simulink model "MemMgrForClima"  (R2022b)
settings: Solver=FixedStepDiscrete  StartTime=0.0  StopTime=10.0  FixedStep=0.1  RelTol=1e-3
model workspace (MAT data in the model file):
  MemMgrForClimaNvArray : Simulink.Signal (value not decoded)
  MemMgrForClimaNvInit : Simulink.Parameter (value not decoded)
symbols that are objects in the model workspace (value not decoded): MemMgrForClimaNvInit
(part 2 of 2)

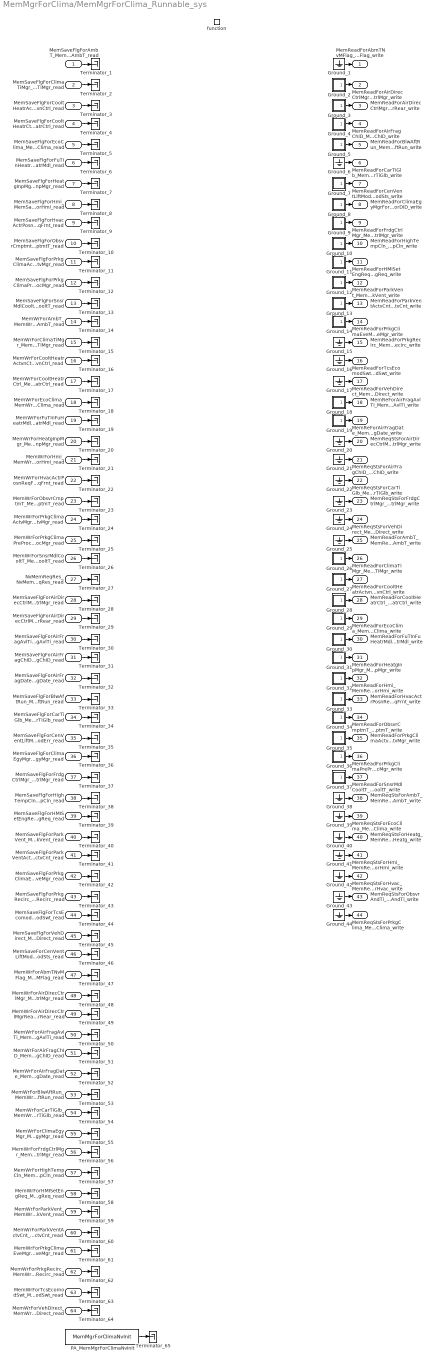
== system MemMgrForClima/MemMgrForClima_Runnable_sys ==
  MemSaveFlgForAmbT_MemSaveFlgForAmbT_read : Inport
  MemSaveFlgForClimaTiMgr_MemSaveFlgForClimaTiMgr_read : Inport  Port=2
  MemSaveFlgForCooltHeatrActvnCtrl_MemSaveFlgForCooltHeatrActvnCtrl_read : Inport  Port=3
  MemSaveFlgForCooltHeatrCtrl_MemSaveFlgForCooltHeatrCtrl_read : Inport  Port=4
  MemSaveFlgForEcoClima_MemSaveFlgForEcoClima_read : Inport  Port=5
  MemSaveFlgForFuTInHeatrMdl_MemSaveFlgForFuTInHeatrMdl_read : Inport  Port=6
  MemSaveFlgForHeatgInpMgr_MemSaveFlgForHeatgInpMgr_read : Inport  Port=7
  MemSaveFlgForHmi_MemSaveFlgForHmi_read : Inport  Port=8
  MemSaveFlgForHvacActrPosnReqFrnt_MemSaveFlgForHvacActrPosnReqFrnt_read : Inport  Port=9
  MemSaveFlgForObsvrCmptmtT_MemSaveFlgForObsvrCmptmtT_read : Inport  Port=10
  MemSaveFlgForPrkgClimaActvMgr_MemSaveFlgForPrkgClimaActvMgr_read : Inport  Port=11
  MemSaveFlgForPrkgClimaPreProcMgr_MemSaveFlgForPrkgClimaPreProcMgr_read : Inport  Port=12
  MemSaveFlgForSnsrMdlCooltT_MemSaveFlgForSnsrMdlCooltT_read : Inport  Port=13
  MemWrForAmbT_MemWrForAmbT_read : Inport  Port=14
  MemWrForClimaTiMgr_MemWrForClimaTiMgr_read : Inport  Port=15
  MemWrForCooltHeatrActvnCtrl_MemWrForCooltHeatrActvnCtrl_read : Inport  Port=16
  MemWrForCooltHeatrCtrl_MemWrForCooltHeatrCtrl_read : Inport  Port=17
  MemWrForEcoClima_MemWrForEcoClima_read : Inport  Port=18
  MemWrForFuTInFuHeatrMdl_MemWrForFuTInFuHeatrMdl_read : Inport  Port=19
  MemWrForHeatgInpMgr_MemWrForHeatgInpMgr_read : Inport  Port=20
  MemWrForHmi_MemWrForHmi_read : Inport  Port=21
  MemWrForHvacActrPosnReqFrnt_MemWrForHvacActrPosnReqFrnt_read : Inport  Port=22
  MemWrForObsvrCmptmT_MemWrForObsvrCmptmT_read : Inport  Port=23
  MemWrForPrkgClimaActvMgr_MemWrForPrkgClimaActvMgr_read : Inport  Port=24
  MemWrForPrkgClimaPreProcMgr_MemWrForPrkgClimaPreProcMgr_read : Inport  Port=25
  MemWrForSnsrMdlCooltT_MemWrForSnsrMdlCooltT_read : Inport  Port=26
  NvMemReqRes_NvMemReqRes_read : Inport  Port=27
  MemSaveFlgForAirDirecCtrlMgr_MemSaveFlgForAirDirecCtrlMgr_read : Inport  Port=28
  MemSaveFlgForAirDirecCtrlMgrRear_MemSaveFlgForAirDirecCtrlMgrRear_read : Inport  Port=29
  MemSaveFlgForAirFragAvlTi_MemSaveFlgForAirFragAvlTi_read : Inport  Port=30
  MemSaveFlgForAirFragChID_MemSaveFlgForAirFragChID_read : Inport  Port=31
  MemSaveFlgForAirFragDate_MemSaveFlgForAirFragDate_read : Inport  Port=32
  MemSaveFlgForBlwAftRun_MemSaveFlgForBlwAftRun_read : Inport  Port=33
  MemSaveFlgForCarTiGlb_MemSaveFlgForCarTiGlb_read : Inport  Port=34
  MemSaveFlgForCenVentLiftModErr_MemSaveFlgForCenVentLiftModErr_read : Inport  Port=35
  MemSaveFlgForClimaEgyMgr_MemSaveFlgForClimaEgyMgr_read : Inport  Port=36
  MemSaveFlgForFrdgCtrlMgr_MemSaveFlgForFrdgCtrlMgr_read : Inport  Port=37
  MemSaveFlgForHighTempCln_MemSaveFlgForHighTempCln_read : Inport  Port=38
  MemSaveFlgForHMISetEngReq_MemSaveFlgForHMISetEngReq_read : Inport  Port=39
  MemSaveFlgForParkVent_MemSaveFlgForParkVent_read : Inport  Port=40
  MemSaveFlgForParkVentActvCnt_MemSaveFlgForParkVentActvCnt_read : Inport  Port=41
  MemSaveFlgForPrkgClimaEveMgr_MemSaveFlgForPrkgClimaEveMgr_read : Inport  Port=42
  MemSaveFlgForPrkgRecirc_MemSaveFlgForPrkgRecirc_read : Inport  Port=43
  MemSaveFlgForTcsEcomodSwt_MemSaveFlgForTcsEcomodSwt_read : Inport  Port=44
  MemSaveFlgForVehDirect_MemSaveFlgForVehDirect_read : Inport  Port=45
  MemSaveForCenVentLiftModSts_MemSaveForCenVentLiftModSts_read : Inport  Port=46
  MemWrForAbmTNvMFlag_MemWrForAbmTNvMFlag_read : Inport  Port=47
  MemWrForAirDirecCtrlMgr_MemWrForAirDirecCtrlMgr_read : Inport  Port=48
  MemWrForAirDirecCtrlMgrRear_MemWrForAirDirecCtrlMgrRear_read : Inport  Port=49
  MemWrForAirFragAvlTi_MemWrForAirFragAvlTi_read : Inport  Port=50
  MemWrForAirFragChID_MemWrForAirFragChID_read : Inport  Port=51
  MemWrForAirFragDate_MemWrForAirFragDate_read : Inport  Port=52
  MemWrForBlwAftRun_MemWrForBlwAftRun_read : Inport  Port=53
  MemWrForCarTiGlb_MemWrForCarTiGlb_read : Inport  Port=54
  MemWrForClimaEgyMgr_MemWrForClimaEgyMgr_read : Inport  Port=55
  MemWrForFrdgCtrlMgr_MemWrForFrdgCtrlMgr_read : Inport  Port=56
  MemWrForHighTempCln_MemWrForHighTempCln_read : Inport  Port=57
  MemWrForHMISetEngReq_MemWrForHMISetEngReq_read : Inport  Port=58
  MemWrForParkVent_MemWrForParkVent_read : Inport  Port=59
  MemWrForParkVentActvCnt_MemWrForParkVentActvCnt_read : Inport  Port=60
  MemWrForPrkgClimaEveMgr_MemWrForPrkgClimaEveMgr_read : Inport  Port=61
  MemWrForPrkgRecirc_MemWrForPrkgRecirc_read : Inport  Port=62
  MemWrForTcsEcomodSwt_MemWrForTcsEcomodSwt_read : Inport  Port=63
  MemWrForVehDirect_MemWrForVehDirect_read : Inport  Port=64
  function : TriggerPort  TriggerType=function-call  VariantControl=(inherit)  SampleTimeType=periodic  SampleTime=-1
  Ground_{1,6,15..17,20..25,38..44} (18 alike) : Ground
  Ground_10 : Subsystem
  Ground_11 : Subsystem
  Ground_12 : Subsystem
  Ground_13 : Subsystem
  Ground_14 : Subsystem
  Ground_18 : Subsystem
  Ground_19 : Subsystem
  Ground_2 : Subsystem
  Ground_26 : Subsystem
  Ground_27 : Subsystem
  Ground_28 : Subsystem
  Ground_29 : Subsystem
  Ground_3 : Subsystem
  Ground_30 : Subsystem
  Ground_31 : Subsystem
  Ground_32 : Subsystem
  Ground_33 : Subsystem
  Ground_34 : Subsystem
  Ground_35 : Subsystem
  Ground_36 : Subsystem
  Ground_37 : Subsystem
  Ground_4 : Subsystem
  Ground_5 : Subsystem
  Ground_7 : Subsystem
  Ground_8 : Subsystem
  Ground_9 : Subsystem
  PA_MemMgrForClimaNvInit : Constant  Value=MemMgrForClimaNvInit
  Terminator_{1..65} (65 alike) : Terminator
  MemReadForAbmTNvMFlag_MemReadForAbmTNvMFlag_write : Outport
      settings: EnsureOutportIsVirtual=on
  MemReadForAirDirecCtrlMgr_MemReadForAirDirecCtrlMgr_write : Outport  Port=2
      settings: EnsureOutportIsVirtual=on
  MemReadForAirDirecCtrlMgrRear_MemReadForAirDirecCtrlMgrRear_write : Outport  Port=3
      settings: EnsureOutportIsVirtual=on
  MemReadForAirFragChID_MemReadForAirFragChID_write : Outport  Port=4
      settings: EnsureOutportIsVirtual=on
  MemReadForBlwAftRun_MemReadForBlwAftRun_write : Outport  Port=5
      settings: EnsureOutportIsVirtual=on
  MemReadForCarTiGlb_MemReadForCarTiGlb_write : Outport  Port=6
      settings: EnsureOutportIsVirtual=on
  MemReadForCenVentLiftModSts_MemReadForCenVentLiftModSts_write : Outport  Port=7
      settings: EnsureOutportIsVirtual=on
  MemReadForClimaEgyMgrForDID_MemReadForClimaEgyMgrForDID_write : Outport  Port=8
      settings: EnsureOutportIsVirtual=on
  MemReadForFrdgCtrlMgr_MemReadForFrdgCtrlMgr_write : Outport  Port=9
      settings: EnsureOutportIsVirtual=on
  MemReadForHighTempCln_MemReadForHighTempCln_write : Outport  Port=10
      settings: EnsureOutportIsVirtual=on
  MemReadForHMISetEngReq_MemReadForHMISetEngReq_write : Outport  Port=11
      settings: EnsureOutportIsVirtual=on
  MemReadForParkVent_MemReadForParkVent_write : Outport  Port=12
      settings: EnsureOutportIsVirtual=on
  MemReadForParkVentActvCnt_MemReadForParkVentActvCnt_write : Outport  Port=13
      settings: EnsureOutportIsVirtual=on
  MemReadForPrkgClimaEveMgr_MemReadForPrkgClimaEveMgr_write : Outport  Port=14
      settings: EnsureOutportIsVirtual=on
  MemReadForPrkgRecirc_MemReadForPrkgRecirc_write : Outport  Port=15
      settings: EnsureOutportIsVirtual=on
  MemReadForTcsEcomodSwt_MemReadForTcsEcomodSwt_write : Outport  Port=16
      settings: EnsureOutportIsVirtual=on
  MemReadForVehDirect_MemReadForVehDirect_write : Outport  Port=17
      settings: EnsureOutportIsVirtual=on
  MemReForAirFragAvlTi_MemReForAirFragAvlTi_write : Outport  Port=18
      settings: EnsureOutportIsVirtual=on
  MemReForAirFragDate_MemReForAirFragDate_write : Outport  Port=19
      settings: EnsureOutportIsVirtual=on
  MemReqStsForAirDirecCtrlMgr_MemReqStsForAirDirecCtrlMgr_write : Outport  Port=20
      settings: EnsureOutportIsVirtual=on
  MemReqStsForAirFragChID_MemReqStsForAirFragChID_write : Outport  Port=21
      settings: EnsureOutportIsVirtual=on
  MemReqStsForCarTiGlb_MemReqStsForCarTiGlb_write : Outport  Port=22
      settings: EnsureOutportIsVirtual=on
  MemReqStsForFrdgCtrlMgr_MemReqStsForFrdgCtrlMgr_write : Outport  Port=23
      settings: EnsureOutportIsVirtual=on
  MemReqStsForVehDirect_MemReqStsForVehDirect_write : Outport  Port=24
      settings: EnsureOutportIsVirtual=on
  MemReadForAmbT_MemReadForAmbT_write : Outport  Port=25
      settings: EnsureOutportIsVirtual=on
  MemReadForClimaTiMgr_MemReadForClimaTiMgr_write : Outport  Port=26
      settings: EnsureOutportIsVirtual=on
  MemReadForCooltHeatrActvnCtrl_MemReadForCooltHeatrActvnCtrl_write : Outport  Port=27
      settings: EnsureOutportIsVirtual=on
  MemReadForCooltHeatrCtrl_MemReadForCooltHeatrCtrl_write : Outport  Port=28
      settings: EnsureOutportIsVirtual=on
  MemReadForEcoClima_MemReadForEcoClima_write : Outport  Port=29
      settings: EnsureOutportIsVirtual=on
  MemReadForFuTInFuHeatrMdl_MemReadForFuTInFuHeatrMdl_write : Outport  Port=30
      settings: EnsureOutportIsVirtual=on
  MemReadForHeatgInpMgr_MemReadForHeatgInpMgr_write : Outport  Port=31
      settings: EnsureOutportIsVirtual=on
  MemReadForHmi_MemReadForHmi_write : Outport  Port=32
      settings: EnsureOutportIsVirtual=on
  MemReadForHvacActrPosnReqFrnt_MemReadForHvacActrPosnReqFrnt_write : Outport  Port=33
      settings: EnsureOutportIsVirtual=on
  MemReadForObsvrCmptmT_MemReadForObsvrCmptmT_write : Outport  Port=34
      settings: EnsureOutportIsVirtual=on
  MemReadForPrkgClimaActvMgr_MemReadForPrkgClimaActvMgr_write : Outport  Port=35
      settings: EnsureOutportIsVirtual=on
  MemReadForPrkgClimaPreProcMgr_MemReadForPrkgClimaPreProcMgr_write : Outport  Port=36
      settings: EnsureOutportIsVirtual=on
  MemReadForSnsrMdlCooltT_MemReadForSnsrMdlCooltT_write : Outport  Port=37
      settings: EnsureOutportIsVirtual=on
  MemReqStsForAmbT_MemReqStsForAmbT_write : Outport  Port=38
      settings: EnsureOutportIsVirtual=on
  MemReqStsForEcoClima_MemReqStsForEcoClima_write : Outport  Port=39
      settings: EnsureOutportIsVirtual=on
  MemReqStsForHeatg_MemReqStsForHeatg_write : Outport  Port=40
      settings: EnsureOutportIsVirtual=on
  MemReqStsForHmi_MemReqStsForHmi_write : Outport  Port=41
      settings: EnsureOutportIsVirtual=on
  MemReqStsForHvac_MemReqStsForHvac_write : Outport  Port=42
      settings: EnsureOutportIsVirtual=on
  MemReqStsForObsvrAndTi_MemReqStsForObsvrAndTi_write : Outport  Port=43
      settings: EnsureOutportIsVirtual=on
  MemReqStsForPrkgClima_MemReqStsForPrkgClima_write : Outport  Port=44
      settings: EnsureOutportIsVirtual=on
  wires:
  MemSaveFlgForAmbT_MemSaveFlgForAmbT_read/1 -> Terminator_1/1
  MemSaveFlgForClimaTiMgr_MemSaveFlgForClimaTiMgr_read/1 -> Terminator_2/1
  MemSaveFlgForCooltHeatrActvnCtrl_MemSaveFlgForCooltHeatrActvnCtrl_read/1 -> Terminator_3/1
  MemSaveFlgForCooltHeatrCtrl_MemSaveFlgForCooltHeatrCtrl_read/1 -> Terminator_4/1
  MemSaveFlgForEcoClima_MemSaveFlgForEcoClima_read/1 -> Terminator_5/1
  MemSaveFlgForFuTInHeatrMdl_MemSaveFlgForFuTInHeatrMdl_read/1 -> Terminator_6/1
  MemSaveFlgForHeatgInpMgr_MemSaveFlgForHeatgInpMgr_read/1 -> Terminator_7/1
  MemSaveFlgForHmi_MemSaveFlgForHmi_read/1 -> Terminator_8/1
  MemSaveFlgForHvacActrPosnReqFrnt_MemSaveFlgForHvacActrPosnReqFrnt_read/1 -> Terminator_9/1
  MemSaveFlgForObsvrCmptmtT_MemSaveFlgForObsvrCmptmtT_read/1 -> Terminator_10/1
  MemSaveFlgForPrkgClimaActvMgr_MemSaveFlgForPrkgClimaActvMgr_read/1 -> Terminator_11/1
  MemSaveFlgForPrkgClimaPreProcMgr_MemSaveFlgForPrkgClimaPreProcMgr_read/1 -> Terminator_12/1
  MemSaveFlgForSnsrMdlCooltT_MemSaveFlgForSnsrMdlCooltT_read/1 -> Terminator_13/1
  MemWrForAmbT_MemWrForAmbT_read/1 -> Terminator_14/1
  MemWrForClimaTiMgr_MemWrForClimaTiMgr_read/1 -> Terminator_15/1
  MemWrForCooltHeatrActvnCtrl_MemWrForCooltHeatrActvnCtrl_read/1 -> Terminator_16/1
  MemWrForCooltHeatrCtrl_MemWrForCooltHeatrCtrl_read/1 -> Terminator_17/1
  MemWrForEcoClima_MemWrForEcoClima_read/1 -> Terminator_18/1
  MemWrForFuTInFuHeatrMdl_MemWrForFuTInFuHeatrMdl_read/1 -> Terminator_19/1
  MemWrForHeatgInpMgr_MemWrForHeatgInpMgr_read/1 -> Terminator_20/1
  MemWrForHmi_MemWrForHmi_read/1 -> Terminator_21/1
  MemWrForHvacActrPosnReqFrnt_MemWrForHvacActrPosnReqFrnt_read/1 -> Terminator_22/1
  MemWrForObsvrCmptmT_MemWrForObsvrCmptmT_read/1 -> Terminator_23/1
  MemWrForPrkgClimaActvMgr_MemWrForPrkgClimaActvMgr_read/1 -> Terminator_24/1
  MemWrForPrkgClimaPreProcMgr_MemWrForPrkgClimaPreProcMgr_read/1 -> Terminator_25/1
  MemWrForSnsrMdlCooltT_MemWrForSnsrMdlCooltT_read/1 -> Terminator_26/1
  NvMemReqRes_NvMemReqRes_read/1 -> Terminator_27/1
  MemSaveFlgForAirDirecCtrlMgr_MemSaveFlgForAirDirecCtrlMgr_read/1 -> Terminator_28/1
  MemSaveFlgForAirDirecCtrlMgrRear_MemSaveFlgForAirDirecCtrlMgrRear_read/1 -> Terminator_29/1
  MemSaveFlgForAirFragAvlTi_MemSaveFlgForAirFragAvlTi_read/1 -> Terminator_30/1
  MemSaveFlgForAirFragChID_MemSaveFlgForAirFragChID_read/1 -> Terminator_31/1
  MemSaveFlgForAirFragDate_MemSaveFlgForAirFragDate_read/1 -> Terminator_32/1
  MemSaveFlgForBlwAftRun_MemSaveFlgForBlwAftRun_read/1 -> Terminator_33/1
  MemSaveFlgForCarTiGlb_MemSaveFlgForCarTiGlb_read/1 -> Terminator_34/1
  MemSaveFlgForCenVentLiftModErr_MemSaveFlgForCenVentLiftModErr_read/1 -> Terminator_35/1
  MemSaveFlgForClimaEgyMgr_MemSaveFlgForClimaEgyMgr_read/1 -> Terminator_36/1
  MemSaveFlgForFrdgCtrlMgr_MemSaveFlgForFrdgCtrlMgr_read/1 -> Terminator_37/1
  MemSaveFlgForHighTempCln_MemSaveFlgForHighTempCln_read/1 -> Terminator_38/1
  MemSaveFlgForHMISetEngReq_MemSaveFlgForHMISetEngReq_read/1 -> Terminator_39/1
  MemSaveFlgForParkVent_MemSaveFlgForParkVent_read/1 -> Terminator_40/1
  MemSaveFlgForParkVentActvCnt_MemSaveFlgForParkVentActvCnt_read/1 -> Terminator_41/1
  MemSaveFlgForPrkgClimaEveMgr_MemSaveFlgForPrkgClimaEveMgr_read/1 -> Terminator_42/1
  MemSaveFlgForPrkgRecirc_MemSaveFlgForPrkgRecirc_read/1 -> Terminator_43/1
  MemSaveFlgForTcsEcomodSwt_MemSaveFlgForTcsEcomodSwt_read/1 -> Terminator_44/1
  MemSaveFlgForVehDirect_MemSaveFlgForVehDirect_read/1 -> Terminator_45/1
  MemSaveForCenVentLiftModSts_MemSaveForCenVentLiftModSts_read/1 -> Terminator_46/1
  MemWrForAbmTNvMFlag_MemWrForAbmTNvMFlag_read/1 -> Terminator_47/1
  MemWrForAirDirecCtrlMgr_MemWrForAirDirecCtrlMgr_read/1 -> Terminator_48/1
  MemWrForAirDirecCtrlMgrRear_MemWrForAirDirecCtrlMgrRear_read/1 -> Terminator_49/1
  MemWrForAirFragAvlTi_MemWrForAirFragAvlTi_read/1 -> Terminator_50/1
  MemWrForAirFragChID_MemWrForAirFragChID_read/1 -> Terminator_51/1
  MemWrForAirFragDate_MemWrForAirFragDate_read/1 -> Terminator_52/1
  MemWrForBlwAftRun_MemWrForBlwAftRun_read/1 -> Terminator_53/1
  MemWrForCarTiGlb_MemWrForCarTiGlb_read/1 -> Terminator_54/1
  MemWrForClimaEgyMgr_MemWrForClimaEgyMgr_read/1 -> Terminator_55/1
  MemWrForFrdgCtrlMgr_MemWrForFrdgCtrlMgr_read/1 -> Terminator_56/1
  MemWrForHighTempCln_MemWrForHighTempCln_read/1 -> Terminator_57/1
  MemWrForHMISetEngReq_MemWrForHMISetEngReq_read/1 -> Terminator_58/1
  MemWrForParkVent_MemWrForParkVent_read/1 -> Terminator_59/1
  MemWrForParkVentActvCnt_MemWrForParkVentActvCnt_read/1 -> Terminator_60/1
  MemWrForPrkgClimaEveMgr_MemWrForPrkgClimaEveMgr_read/1 -> Terminator_61/1
  MemWrForPrkgRecirc_MemWrForPrkgRecirc_read/1 -> Terminator_62/1
  MemWrForTcsEcomodSwt_MemWrForTcsEcomodSwt_read/1 -> Terminator_63/1
  MemWrForVehDirect_MemWrForVehDirect_read/1 -> Terminator_64/1
  PA_MemMgrForClimaNvInit/1 -> Terminator_65/1
  Ground_1/1 -> MemReadForAbmTNvMFlag_MemReadForAbmTNvMFlag_write/1
  Ground_2/1(Out1) -> MemReadForAirDirecCtrlMgr_MemReadForAirDirecCtrlMgr_write/1
  Ground_3/1(Out1) -> MemReadForAirDirecCtrlMgrRear_MemReadForAirDirecCtrlMgrRear_write/1
  Ground_4/1(Out1) -> MemReadForAirFragChID_MemReadForAirFragChID_write/1
  Ground_5/1(Out1) -> MemReadForBlwAftRun_MemReadForBlwAftRun_write/1
  Ground_6/1 -> MemReadForCarTiGlb_MemReadForCarTiGlb_write/1
  Ground_7/1(Out1) -> MemReadForCenVentLiftModSts_MemReadForCenVentLiftModSts_write/1
  Ground_8/1(Out1) -> MemReadForClimaEgyMgrForDID_MemReadForClimaEgyMgrForDID_write/1
  Ground_9/1(Out1) -> MemReadForFrdgCtrlMgr_MemReadForFrdgCtrlMgr_write/1
  Ground_10/1(Out1) -> MemReadForHighTempCln_MemReadForHighTempCln_write/1
  Ground_11/1(Out1) -> MemReadForHMISetEngReq_MemReadForHMISetEngReq_write/1
  Ground_12/1(Out1) -> MemReadForParkVent_MemReadForParkVent_write/1
  Ground_13/1(Out1) -> MemReadForParkVentActvCnt_MemReadForParkVentActvCnt_write/1
  Ground_14/1(Out1) -> MemReadForPrkgClimaEveMgr_MemReadForPrkgClimaEveMgr_write/1
  Ground_15/1 -> MemReadForPrkgRecirc_MemReadForPrkgRecirc_write/1
  Ground_16/1 -> MemReadForTcsEcomodSwt_MemReadForTcsEcomodSwt_write/1
  Ground_17/1 -> MemReadForVehDirect_MemReadForVehDirect_write/1
  Ground_18/1(Out1) -> MemReForAirFragAvlTi_MemReForAirFragAvlTi_write/1
  Ground_19/1(Out1) -> MemReForAirFragDate_MemReForAirFragDate_write/1
  Ground_20/1 -> MemReqStsForAirDirecCtrlMgr_MemReqStsForAirDirecCtrlMgr_write/1
  Ground_21/1 -> MemReqStsForAirFragChID_MemReqStsForAirFragChID_write/1
  Ground_22/1 -> MemReqStsForCarTiGlb_MemReqStsForCarTiGlb_write/1
  Ground_23/1 -> MemReqStsForFrdgCtrlMgr_MemReqStsForFrdgCtrlMgr_write/1
  Ground_24/1 -> MemReqStsForVehDirect_MemReqStsForVehDirect_write/1
  Ground_25/1 -> MemReadForAmbT_MemReadForAmbT_write/1
  Ground_26/1(Out1) -> MemReadForClimaTiMgr_MemReadForClimaTiMgr_write/1
  Ground_27/1(Out1) -> MemReadForCooltHeatrActvnCtrl_MemReadForCooltHeatrActvnCtrl_write/1
  Ground_28/1(Out1) -> MemReadForCooltHeatrCtrl_MemReadForCooltHeatrCtrl_write/1
  Ground_29/1(Out1) -> MemReadForEcoClima_MemReadForEcoClima_write/1
  Ground_30/1(Out1) -> MemReadForFuTInFuHeatrMdl_MemReadForFuTInFuHeatrMdl_write/1
  Ground_31/1(Out1) -> MemReadForHeatgInpMgr_MemReadForHeatgInpMgr_write/1
  Ground_32/1(Out1) -> MemReadForHmi_MemReadForHmi_write/1
  Ground_33/1(Out1) -> MemReadForHvacActrPosnReqFrnt_MemReadForHvacActrPosnReqFrnt_write/1
  Ground_34/1(Out1) -> MemReadForObsvrCmptmT_MemReadForObsvrCmptmT_write/1
  Ground_35/1(Out1) -> MemReadForPrkgClimaActvMgr_MemReadForPrkgClimaActvMgr_write/1
  Ground_36/1(Out1) -> MemReadForPrkgClimaPreProcMgr_MemReadForPrkgClimaPreProcMgr_write/1
  Ground_37/1(Out1) -> MemReadForSnsrMdlCooltT_MemReadForSnsrMdlCooltT_write/1
  Ground_38/1 -> MemReqStsForAmbT_MemReqStsForAmbT_write/1
  Ground_39/1 -> MemReqStsForEcoClima_MemReqStsForEcoClima_write/1
  Ground_40/1 -> MemReqStsForHeatg_MemReqStsForHeatg_write/1
  Ground_41/1 -> MemReqStsForHmi_MemReqStsForHmi_write/1
  Ground_42/1 -> MemReqStsForHvac_MemReqStsForHvac_write/1
  Ground_43/1 -> MemReqStsForObsvrAndTi_MemReqStsForObsvrAndTi_write/1
  Ground_44/1 -> MemReqStsForPrkgClima_MemReqStsForPrkgClima_write/1

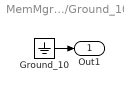
== system MemMgrForClima/MemMgrForClima_Init/Ground_10 ==
  Ground_10 : Ground
  Out1 : Outport  OutDataTypeStr=Bus: MemRecForObsvrCmptmT  PortDimensions=1
  wires:
  Ground_10/1 -> Out1/1

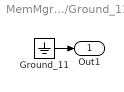
== system MemMgrForClima/MemMgrForClima_Init/Ground_11 ==
  Ground_11 : Ground
  Out1 : Outport  OutDataTypeStr=Bus: MemRecForPrkgClimaActvMgr  PortDimensions=1
  wires:
  Ground_11/1 -> Out1/1

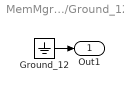
== system MemMgrForClima/MemMgrForClima_Init/Ground_12 ==
  Ground_12 : Ground
  Out1 : Outport  OutDataTypeStr=Bus: MemRecForPrkgClimaPreProcMgr  PortDimensions=1
  wires:
  Ground_12/1 -> Out1/1

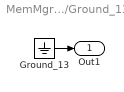
== system MemMgrForClima/MemMgrForClima_Init/Ground_13 ==
  Ground_13 : Ground
  Out1 : Outport  OutDataTypeStr=Bus: MemRecForSnsrMdlCooltT  PortDimensions=1
  wires:
  Ground_13/1 -> Out1/1

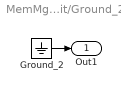
== system MemMgrForClima/MemMgrForClima_Init/Ground_2 ==
  Ground_2 : Ground
  Out1 : Outport  OutDataTypeStr=Bus: MemRecForClimaTiMgr  PortDimensions=1
  wires:
  Ground_2/1 -> Out1/1

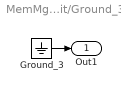
== system MemMgrForClima/MemMgrForClima_Init/Ground_3 ==
  Ground_3 : Ground
  Out1 : Outport  OutDataTypeStr=Bus: MemRecForCooltHeatrActvnCtrl  PortDimensions=1
  wires:
  Ground_3/1 -> Out1/1

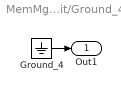
== system MemMgrForClima/MemMgrForClima_Init/Ground_4 ==
  Ground_4 : Ground
  Out1 : Outport  OutDataTypeStr=Bus: MemRecForCooltHeatrCtrl  PortDimensions=1
  wires:
  Ground_4/1 -> Out1/1

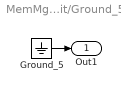
== system MemMgrForClima/MemMgrForClima_Init/Ground_5 ==
  Ground_5 : Ground
  Out1 : Outport  OutDataTypeStr=Bus: MemWrForEcoClima  PortDimensions=1
  wires:
  Ground_5/1 -> Out1/1

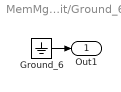
== system MemMgrForClima/MemMgrForClima_Init/Ground_6 ==
  Ground_6 : Ground
  Out1 : Outport  OutDataTypeStr=Bus: MemRecForFuTInFuHeatrMdl  PortDimensions=1
  wires:
  Ground_6/1 -> Out1/1

== system MemMgrForClima/MemMgrForClima_Init/Ground_7 ==
  Ground_7 : Ground
  Out1 : Outport  OutDataTypeStr=Bus: MemRecForHeatgInpMgr  PortDimensions=1
  wires:
  Ground_7/1 -> Out1/1

== system MemMgrForClima/MemMgrForClima_Init/Ground_8 ==
  Ground_8 : Ground
  Out1 : Outport  OutDataTypeStr=Bus: MemRecForHmi  PortDimensions=1
  wires:
  Ground_8/1 -> Out1/1

== system MemMgrForClima/MemMgrForClima_Init/Ground_9 ==
  Ground_9 : Ground
  Out1 : Outport  OutDataTypeStr=Bus: MemRecForHvacActrPosnReqFrnt  PortDimensions=1
  wires:
  Ground_9/1 -> Out1/1

== system MemMgrForClima/MemMgrForClima_Runnable_sys/Ground_10 ==
  Ground_10 : Ground
  Out1 : Outport  OutDataTypeStr=Bus: MemRecForHighTempCln  PortDimensions=1
  wires:
  Ground_10/1 -> Out1/1

== system MemMgrForClima/MemMgrForClima_Runnable_sys/Ground_11 ==
  Ground_11 : Ground
  Out1 : Outport  OutDataTypeStr=Bus: MemRecForHMISetEngReq  PortDimensions=1
  wires:
  Ground_11/1 -> Out1/1

== system MemMgrForClima/MemMgrForClima_Runnable_sys/Ground_12 ==
  Ground_12 : Ground
  Out1 : Outport  OutDataTypeStr=Bus: MemRecForParkVent  PortDimensions=1
  wires:
  Ground_12/1 -> Out1/1

== system MemMgrForClima/MemMgrForClima_Runnable_sys/Ground_13 ==
  Ground_13 : Ground
  Out1 : Outport  OutDataTypeStr=Bus: MemRecForParkVentCnt  PortDimensions=1
  wires:
  Ground_13/1 -> Out1/1

== system MemMgrForClima/MemMgrForClima_Runnable_sys/Ground_14 ==
  Ground_14 : Ground
  Out1 : Outport  OutDataTypeStr=Bus: MemRecForPrkgClimaEveMgr  PortDimensions=1
  wires:
  Ground_14/1 -> Out1/1

== system MemMgrForClima/MemMgrForClima_Runnable_sys/Ground_18 ==
  Ground_18 : Ground
  Out1 : Outport  OutDataTypeStr=Bus: MemReqForAirFragAvlTi  PortDimensions=1
  wires:
  Ground_18/1 -> Out1/1

== system MemMgrForClima/MemMgrForClima_Runnable_sys/Ground_19 ==
  Ground_19 : Ground
  Out1 : Outport  OutDataTypeStr=Bus: MemWrForAirFragDate  PortDimensions=1
  wires:
  Ground_19/1 -> Out1/1

== system MemMgrForClima/MemMgrForClima_Runnable_sys/Ground_2 ==
  Ground_2 : Ground
  Out1 : Outport  OutDataTypeStr=Bus: MemRecForAirDirecCtrlMgr  PortDimensions=1
  wires:
  Ground_2/1 -> Out1/1

== system MemMgrForClima/MemMgrForClima_Runnable_sys/Ground_26 ==
  Ground_26 : Ground
  Out1 : Outport  OutDataTypeStr=Bus: MemRecForClimaTiMgr  PortDimensions=1
  wires:
  Ground_26/1 -> Out1/1

== system MemMgrForClima/MemMgrForClima_Runnable_sys/Ground_27 ==
  Ground_27 : Ground
  Out1 : Outport  OutDataTypeStr=Bus: MemRecForCooltHeatrActvnCtrl  PortDimensions=1
  wires:
  Ground_27/1 -> Out1/1

== system MemMgrForClima/MemMgrForClima_Runnable_sys/Ground_28 ==
  Ground_28 : Ground
  Out1 : Outport  OutDataTypeStr=Bus: MemRecForCooltHeatrCtrl  PortDimensions=1
  wires:
  Ground_28/1 -> Out1/1

== system MemMgrForClima/MemMgrForClima_Runnable_sys/Ground_29 ==
  Ground_29 : Ground
  Out1 : Outport  OutDataTypeStr=Bus: MemWrForEcoClima  PortDimensions=1
  wires:
  Ground_29/1 -> Out1/1

== system MemMgrForClima/MemMgrForClima_Runnable_sys/Ground_3 ==
  Ground_3 : Ground
  Out1 : Outport  OutDataTypeStr=Bus: MemRecForAirDirecCtrlMgrRear  PortDimensions=1
  wires:
  Ground_3/1 -> Out1/1

== system MemMgrForClima/MemMgrForClima_Runnable_sys/Ground_30 ==
  Ground_30 : Ground
  Out1 : Outport  OutDataTypeStr=Bus: MemRecForFuTInFuHeatrMdl  PortDimensions=1
  wires:
  Ground_30/1 -> Out1/1

== system MemMgrForClima/MemMgrForClima_Runnable_sys/Ground_31 ==
  Ground_31 : Ground
  Out1 : Outport  OutDataTypeStr=Bus: MemRecForHeatgInpMgr  PortDimensions=1
  wires:
  Ground_31/1 -> Out1/1

== system MemMgrForClima/MemMgrForClima_Runnable_sys/Ground_32 ==
  Ground_32 : Ground
  Out1 : Outport  OutDataTypeStr=Bus: MemRecForHmi  PortDimensions=1
  wires:
  Ground_32/1 -> Out1/1

== system MemMgrForClima/MemMgrForClima_Runnable_sys/Ground_33 ==
  Ground_33 : Ground
  Out1 : Outport  OutDataTypeStr=Bus: MemRecForHvacActrPosnReqFrnt  PortDimensions=1
  wires:
  Ground_33/1 -> Out1/1

== system MemMgrForClima/MemMgrForClima_Runnable_sys/Ground_34 ==
  Ground_34 : Ground
  Out1 : Outport  OutDataTypeStr=Bus: MemRecForObsvrCmptmT  PortDimensions=1
  wires:
  Ground_34/1 -> Out1/1

== system MemMgrForClima/MemMgrForClima_Runnable_sys/Ground_35 ==
  Ground_35 : Ground
  Out1 : Outport  OutDataTypeStr=Bus: MemRecForPrkgClimaActvMgr  PortDimensions=1
  wires:
  Ground_35/1 -> Out1/1

== system MemMgrForClima/MemMgrForClima_Runnable_sys/Ground_36 ==
  Ground_36 : Ground
  Out1 : Outport  OutDataTypeStr=Bus: MemRecForPrkgClimaPreProcMgr  PortDimensions=1
  wires:
  Ground_36/1 -> Out1/1

== system MemMgrForClima/MemMgrForClima_Runnable_sys/Ground_37 ==
  Ground_37 : Ground
  Out1 : Outport  OutDataTypeStr=Bus: MemRecForSnsrMdlCooltT  PortDimensions=1
  wires:
  Ground_37/1 -> Out1/1

== system MemMgrForClima/MemMgrForClima_Runnable_sys/Ground_4 ==
  Ground_4 : Ground
  Out1 : Outport  OutDataTypeStr=Bus: MemReqForAirFragChID  PortDimensions=1
  wires:
  Ground_4/1 -> Out1/1

== system MemMgrForClima/MemMgrForClima_Runnable_sys/Ground_5 ==
  Ground_5 : Ground
  Out1 : Outport  OutDataTypeStr=Bus: MemRecForBlwAftRun  PortDimensions=1
  wires:
  Ground_5/1 -> Out1/1

== system MemMgrForClima/MemMgrForClima_Runnable_sys/Ground_7 ==
  Ground_7 : Ground
  Out1 : Outport  OutDataTypeStr=Bus: MemSaveLiftMod  PortDimensions=1
  wires:
  Ground_7/1 -> Out1/1

== system MemMgrForClima/MemMgrForClima_Runnable_sys/Ground_8 ==
  Ground_8 : Ground
  Out1 : Outport  OutDataTypeStr=Bus: MemRecForClimaEgyMgr  PortDimensions=1
  wires:
  Ground_8/1 -> Out1/1

== system MemMgrForClima/MemMgrForClima_Runnable_sys/Ground_9 ==
  Ground_9 : Ground
  Out1 : Outport  OutDataTypeStr=Bus: MemRecForFrdgCtrlMgr  PortDimensions=1
  wires:
  Ground_9/1 -> Out1/1
